simulink model "TSR_Scenario_005_cs"  (Simulink 7.6)
settings: Solver=ode5  SolverMode=SingleTasking  StartTime=0.0  StopTime=inf  FixedStep=1/100  RelTol=1e-3
PreLoadFcn:
  | check_NUTS_defined;
  | 
  | assignin('base',['model_closed_' bdroot],false);
  | 
  | addpath('../includes_TSR/includes_TSR');
  | 
  | switch version('-release')
  | case {'2010a', '2010b', '2011a'}
  | addpath('../includes_TSR/ML2010b');
  | otherwise
  | addpath('../includes_TSR/ML2011b');
  | end
InitFcn:
  | PreScanExperimentInitFcn(bdroot)
StopFcn:
  | PreScanExperimentStopFcn(bdroot)
undefined symbols (not defined in the file): DiscIntNeverNeededParam, InitImageOutputDir, x_ini_VRML, y_ini_VRML, z_ini_VRML
libraries: PreScanSimulinkLibrary x97, simulink x59, PreScanUsefulBlocks x33, simulink_extras x30, simulink_need_slupdate x1
(part 23 of 26)

== system TSR_Scenario_005_cs/Sphere_1/Dynamics_Empty/MuxState ==
  note: mode: Dynamics
  note: mode: Positioning
  note: mode: Dynamics
      | VelVEHICLE
      | wrt VEH
  note: AnglesVEHICLE
      | wrtGLOB
  note: For state mode 'Dynamic' positions
      | are not taken into account, as they
      | are computed by integrating velocity.
      | Click annotation for Help.
  x [m] : Inport  SampleTime=TimeStep
  y [m] : Inport  Port=2  SampleTime=TimeStep
  z [m] : Inport  Port=3  SampleTime=TimeStep
  dx [m/s] : Inport  Port=4  SampleTime=TimeStep
  dy [m/s] : Inport  Port=5  SampleTime=TimeStep
  dz [m/s] : Inport  Port=6  SampleTime=TimeStep
  R [rad] : Inport  Port=7  SampleTime=TimeStep
  P [rad] : Inport  Port=8  SampleTime=TimeStep
  Y [rad] : Inport  Port=9  SampleTime=TimeStep
  Constant : Constant  DisableCoverage=on  Value=positioningmode
  Discrete-Time Integrator : DiscreteIntegrator  SampleTime=TimeStep  ICPrevOutput=DiscIntNeverNeededParam  ICPrevScaledInput=DiscIntNeverNeededParam
  Ground : Ground
  Ground1 : Ground
  Mux1 : Mux  Inputs=[3 3]
  Mux2 : Mux  Inputs=3
  Mux3 : Mux  Inputs=3
  Mux4 : Mux  Inputs=3
  Mux5 : Mux  Inputs=[3 3]
  Mux6 : Mux  Inputs=[6 3]
  Switch : Switch  Threshold=0.5
  Transformation_BODY2GLOBAL : PreScanSimulinkLibrary/Simple_Vehicle_Dynamics/Chassis/MuxDynState/Transformation_BODY2GLOBAL  SystemSampleTime=-1
  mux3 : Mux  Inputs=3
  state : Outport
  wires:
  Constant/1 -> Switch/2
  Mux6/1 -> state/1
  Mux4/1 -> Mux1/1
  Mux5/1 -> Switch/3
  Mux1/1 -> Switch/1
  Switch/1 -> Mux6/1  "PosVeh wrt  GLOB"
  P [rad]/1 -> Mux3/2
  R [rad]/1 -> Mux3/1
  dz [m/s]/1 -> Mux2/3
  dy [m/s]/1 -> Mux2/2
  dx [m/s]/1 -> Mux2/1
  z [m]/1 -> Mux4/3
  y [m]/1 -> Mux4/2
  x [m]/1 -> Mux4/1
  Ground1/1 -> mux3/2  "pitch"
  Y [rad]/1 -> mux3/3, Mux3/3  "yaw"
  Mux3/1 -> Mux6/2
  Transformation_BODY2GLOBAL/1 -> Discrete-Time Integrator/1, Mux5/2  "VelVEH wrt  GLOB"
  mux3/1 -> Transformation_BODY2GLOBAL/2
  Ground/1 -> mux3/1  "roll"
  Mux2/1 -> Mux1/2, Transformation_BODY2GLOBAL/1
  Discrete-Time Integrator/1 -> Mux5/1  "PosVEH wrt  GLOB"

== system TSR_Scenario_005_cs/Sphere_1/SELF_Demux/compas converter ==
  rotz [rad] : Inport
  cart2compas : Subsystem
  rad2deg : Gain  Gain=180/pi
  heading [deg] : Outport
  wires:
  cart2compas/1(heading [deg]) -> heading [deg]/1
  rotz [rad]/1 -> rad2deg/1
  rad2deg/1 -> cart2compas/1(Angle [deg])

== system TSR_Scenario_005_cs/Toyota_Previa_1/Dynamics_Empty/MuxState ==
  note: mode: Dynamics
  note: mode: Positioning
  note: mode: Dynamics
      | VelVEHICLE
      | wrt VEH
  note: AnglesVEHICLE
      | wrtGLOB
  note: For state mode 'Dynamic' positions
      | are not taken into account, as they
      | are computed by integrating velocity.
      | Click annotation for Help.
  x [m] : Inport  SampleTime=TimeStep
  y [m] : Inport  Port=2  SampleTime=TimeStep
  z [m] : Inport  Port=3  SampleTime=TimeStep
  dx [m/s] : Inport  Port=4  SampleTime=TimeStep
  dy [m/s] : Inport  Port=5  SampleTime=TimeStep
  dz [m/s] : Inport  Port=6  SampleTime=TimeStep
  R [rad] : Inport  Port=7  SampleTime=TimeStep
  P [rad] : Inport  Port=8  SampleTime=TimeStep
  Y [rad] : Inport  Port=9  SampleTime=TimeStep
  Constant : Constant  DisableCoverage=on  Value=positioningmode
  Discrete-Time Integrator : DiscreteIntegrator  SampleTime=TimeStep  ICPrevOutput=DiscIntNeverNeededParam  ICPrevScaledInput=DiscIntNeverNeededParam
  Ground : Ground
  Ground1 : Ground
  Mux1 : Mux  Inputs=[3 3]
  Mux2 : Mux  Inputs=3
  Mux3 : Mux  Inputs=3
  Mux4 : Mux  Inputs=3
  Mux5 : Mux  Inputs=[3 3]
  Mux6 : Mux  Inputs=[6 3]
  Switch : Switch  Threshold=0.5
  Transformation_BODY2GLOBAL : PreScanSimulinkLibrary/Simple_Vehicle_Dynamics/Chassis/MuxDynState/Transformation_BODY2GLOBAL  SystemSampleTime=-1
  mux3 : Mux  Inputs=3
  state : Outport
  wires:
  Constant/1 -> Switch/2
  Mux6/1 -> state/1
  Mux4/1 -> Mux1/1
  Mux5/1 -> Switch/3
  Mux1/1 -> Switch/1
  Switch/1 -> Mux6/1  "PosVeh wrt  GLOB"
  P [rad]/1 -> Mux3/2
  R [rad]/1 -> Mux3/1
  dz [m/s]/1 -> Mux2/3
  dy [m/s]/1 -> Mux2/2
  dx [m/s]/1 -> Mux2/1
  z [m]/1 -> Mux4/3
  y [m]/1 -> Mux4/2
  x [m]/1 -> Mux4/1
  Ground1/1 -> mux3/2  "pitch"
  Y [rad]/1 -> mux3/3, Mux3/3  "yaw"
  Mux3/1 -> Mux6/2
  Transformation_BODY2GLOBAL/1 -> Discrete-Time Integrator/1, Mux5/2  "VelVEH wrt  GLOB"
  mux3/1 -> Transformation_BODY2GLOBAL/2
  Ground/1 -> mux3/1  "roll"
  Mux2/1 -> Mux1/2, Transformation_BODY2GLOBAL/1
  Discrete-Time Integrator/1 -> Mux5/1  "PosVEH wrt  GLOB"

== system TSR_Scenario_005_cs/Toyota_Previa_1/SELF_Demux/compas converter ==
  rotz [rad] : Inport
  cart2compas : Subsystem
  rad2deg : Gain  Gain=180/pi
  heading [deg] : Outport
  wires:
  cart2compas/1(heading [deg]) -> heading [deg]/1
  rotz [rad]/1 -> rad2deg/1
  rad2deg/1 -> cart2compas/1(Angle [deg])

== system TSR_Scenario_005_cs/Toyota_Previa_2/Dynamics_Empty/MuxState ==
  note: mode: Dynamics
  note: mode: Positioning
  note: mode: Dynamics
      | VelVEHICLE
      | wrt VEH
  note: AnglesVEHICLE
      | wrtGLOB
  note: For state mode 'Dynamic' positions
      | are not taken into account, as they
      | are computed by integrating velocity.
      | Click annotation for Help.
  x [m] : Inport  SampleTime=TimeStep
  y [m] : Inport  Port=2  SampleTime=TimeStep
  z [m] : Inport  Port=3  SampleTime=TimeStep
  dx [m/s] : Inport  Port=4  SampleTime=TimeStep
  dy [m/s] : Inport  Port=5  SampleTime=TimeStep
  dz [m/s] : Inport  Port=6  SampleTime=TimeStep
  R [rad] : Inport  Port=7  SampleTime=TimeStep
  P [rad] : Inport  Port=8  SampleTime=TimeStep
  Y [rad] : Inport  Port=9  SampleTime=TimeStep
  Constant : Constant  DisableCoverage=on  Value=positioningmode
  Discrete-Time Integrator : DiscreteIntegrator  SampleTime=TimeStep  ICPrevOutput=DiscIntNeverNeededParam  ICPrevScaledInput=DiscIntNeverNeededParam
  Ground : Ground
  Ground1 : Ground
  Mux1 : Mux  Inputs=[3 3]
  Mux2 : Mux  Inputs=3
  Mux3 : Mux  Inputs=3
  Mux4 : Mux  Inputs=3
  Mux5 : Mux  Inputs=[3 3]
  Mux6 : Mux  Inputs=[6 3]
  Switch : Switch  Threshold=0.5
  Transformation_BODY2GLOBAL : PreScanSimulinkLibrary/Simple_Vehicle_Dynamics/Chassis/MuxDynState/Transformation_BODY2GLOBAL  SystemSampleTime=-1
  mux3 : Mux  Inputs=3
  state : Outport
  wires:
  Constant/1 -> Switch/2
  Mux6/1 -> state/1
  Mux4/1 -> Mux1/1
  Mux5/1 -> Switch/3
  Mux1/1 -> Switch/1
  Switch/1 -> Mux6/1  "PosVeh wrt  GLOB"
  P [rad]/1 -> Mux3/2
  R [rad]/1 -> Mux3/1
  dz [m/s]/1 -> Mux2/3
  dy [m/s]/1 -> Mux2/2
  dx [m/s]/1 -> Mux2/1
  z [m]/1 -> Mux4/3
  y [m]/1 -> Mux4/2
  x [m]/1 -> Mux4/1
  Ground1/1 -> mux3/2  "pitch"
  Y [rad]/1 -> mux3/3, Mux3/3  "yaw"
  Mux3/1 -> Mux6/2
  Transformation_BODY2GLOBAL/1 -> Discrete-Time Integrator/1, Mux5/2  "VelVEH wrt  GLOB"
  mux3/1 -> Transformation_BODY2GLOBAL/2
  Ground/1 -> mux3/1  "roll"
  Mux2/1 -> Mux1/2, Transformation_BODY2GLOBAL/1
  Discrete-Time Integrator/1 -> Mux5/1  "PosVEH wrt  GLOB"

== system TSR_Scenario_005_cs/Toyota_Previa_2/SELF_Demux/compas converter ==
  rotz [rad] : Inport
  cart2compas : Subsystem
  rad2deg : Gain  Gain=180/pi
  heading [deg] : Outport
  wires:
  cart2compas/1(heading [deg]) -> heading [deg]/1
  rotz [rad]/1 -> rad2deg/1
  rad2deg/1 -> cart2compas/1(Angle [deg])

== system TSR_Scenario_005_cs/Toyota_Previa_3/Dynamics_Empty/MuxState ==
  note: mode: Dynamics
  note: mode: Positioning
  note: mode: Dynamics
      | VelVEHICLE
      | wrt VEH
  note: AnglesVEHICLE
      | wrtGLOB
  note: For state mode 'Dynamic' positions
      | are not taken into account, as they
      | are computed by integrating velocity.
      | Click annotation for Help.
  x [m] : Inport  SampleTime=TimeStep
  y [m] : Inport  Port=2  SampleTime=TimeStep
  z [m] : Inport  Port=3  SampleTime=TimeStep
  dx [m/s] : Inport  Port=4  SampleTime=TimeStep
  dy [m/s] : Inport  Port=5  SampleTime=TimeStep
  dz [m/s] : Inport  Port=6  SampleTime=TimeStep
  R [rad] : Inport  Port=7  SampleTime=TimeStep
  P [rad] : Inport  Port=8  SampleTime=TimeStep
  Y [rad] : Inport  Port=9  SampleTime=TimeStep
  Constant : Constant  DisableCoverage=on  Value=positioningmode
  Discrete-Time Integrator : DiscreteIntegrator  SampleTime=TimeStep  ICPrevOutput=DiscIntNeverNeededParam  ICPrevScaledInput=DiscIntNeverNeededParam
  Ground : Ground
  Ground1 : Ground
  Mux1 : Mux  Inputs=[3 3]
  Mux2 : Mux  Inputs=3
  Mux3 : Mux  Inputs=3
  Mux4 : Mux  Inputs=3
  Mux5 : Mux  Inputs=[3 3]
  Mux6 : Mux  Inputs=[6 3]
  Switch : Switch  Threshold=0.5
  Transformation_BODY2GLOBAL : PreScanSimulinkLibrary/Simple_Vehicle_Dynamics/Chassis/MuxDynState/Transformation_BODY2GLOBAL  SystemSampleTime=-1
  mux3 : Mux  Inputs=3
  state : Outport
  wires:
  Constant/1 -> Switch/2
  Mux6/1 -> state/1
  Mux4/1 -> Mux1/1
  Mux5/1 -> Switch/3
  Mux1/1 -> Switch/1
  Switch/1 -> Mux6/1  "PosVeh wrt  GLOB"
  P [rad]/1 -> Mux3/2
  R [rad]/1 -> Mux3/1
  dz [m/s]/1 -> Mux2/3
  dy [m/s]/1 -> Mux2/2
  dx [m/s]/1 -> Mux2/1
  z [m]/1 -> Mux4/3
  y [m]/1 -> Mux4/2
  x [m]/1 -> Mux4/1
  Ground1/1 -> mux3/2  "pitch"
  Y [rad]/1 -> mux3/3, Mux3/3  "yaw"
  Mux3/1 -> Mux6/2
  Transformation_BODY2GLOBAL/1 -> Discrete-Time Integrator/1, Mux5/2  "VelVEH wrt  GLOB"
  mux3/1 -> Transformation_BODY2GLOBAL/2
  Ground/1 -> mux3/1  "roll"
  Mux2/1 -> Mux1/2, Transformation_BODY2GLOBAL/1
  Discrete-Time Integrator/1 -> Mux5/1  "PosVEH wrt  GLOB"

== system TSR_Scenario_005_cs/Toyota_Previa_3/SELF_Demux/compas converter ==
  rotz [rad] : Inport
  cart2compas : Subsystem
  rad2deg : Gain  Gain=180/pi
  heading [deg] : Outport
  wires:
  cart2compas/1(heading [deg]) -> heading [deg]/1
  rotz [rad]/1 -> rad2deg/1
  rad2deg/1 -> cart2compas/1(Angle [deg])

== system TSR_Scenario_005_cs/Toyota_Previa_4/Dynamics_Empty/MuxState ==
  note: mode: Dynamics
  note: mode: Positioning
  note: mode: Dynamics
      | VelVEHICLE
      | wrt VEH
  note: AnglesVEHICLE
      | wrtGLOB
  note: For state mode 'Dynamic' positions
      | are not taken into account, as they
      | are computed by integrating velocity.
      | Click annotation for Help.
  x [m] : Inport  SampleTime=TimeStep
  y [m] : Inport  Port=2  SampleTime=TimeStep
  z [m] : Inport  Port=3  SampleTime=TimeStep
  dx [m/s] : Inport  Port=4  SampleTime=TimeStep
  dy [m/s] : Inport  Port=5  SampleTime=TimeStep
  dz [m/s] : Inport  Port=6  SampleTime=TimeStep
  R [rad] : Inport  Port=7  SampleTime=TimeStep
  P [rad] : Inport  Port=8  SampleTime=TimeStep
  Y [rad] : Inport  Port=9  SampleTime=TimeStep
  Constant : Constant  DisableCoverage=on  Value=positioningmode
  Discrete-Time Integrator : DiscreteIntegrator  SampleTime=TimeStep  ICPrevOutput=DiscIntNeverNeededParam  ICPrevScaledInput=DiscIntNeverNeededParam
  Ground : Ground
  Ground1 : Ground
  Mux1 : Mux  Inputs=[3 3]
  Mux2 : Mux  Inputs=3
  Mux3 : Mux  Inputs=3
  Mux4 : Mux  Inputs=3
  Mux5 : Mux  Inputs=[3 3]
  Mux6 : Mux  Inputs=[6 3]
  Switch : Switch  Threshold=0.5
  Transformation_BODY2GLOBAL : PreScanSimulinkLibrary/Simple_Vehicle_Dynamics/Chassis/MuxDynState/Transformation_BODY2GLOBAL  SystemSampleTime=-1
  mux3 : Mux  Inputs=3
  state : Outport
  wires:
  Constant/1 -> Switch/2
  Mux6/1 -> state/1
  Mux4/1 -> Mux1/1
  Mux5/1 -> Switch/3
  Mux1/1 -> Switch/1
  Switch/1 -> Mux6/1  "PosVeh wrt  GLOB"
  P [rad]/1 -> Mux3/2
  R [rad]/1 -> Mux3/1
  dz [m/s]/1 -> Mux2/3
  dy [m/s]/1 -> Mux2/2
  dx [m/s]/1 -> Mux2/1
  z [m]/1 -> Mux4/3
  y [m]/1 -> Mux4/2
  x [m]/1 -> Mux4/1
  Ground1/1 -> mux3/2  "pitch"
  Y [rad]/1 -> mux3/3, Mux3/3  "yaw"
  Mux3/1 -> Mux6/2
  Transformation_BODY2GLOBAL/1 -> Discrete-Time Integrator/1, Mux5/2  "VelVEH wrt  GLOB"
  mux3/1 -> Transformation_BODY2GLOBAL/2
  Ground/1 -> mux3/1  "roll"
  Mux2/1 -> Mux1/2, Transformation_BODY2GLOBAL/1
  Discrete-Time Integrator/1 -> Mux5/1  "PosVEH wrt  GLOB"

== system TSR_Scenario_005_cs/Toyota_Previa_4/SELF_Demux/compas converter ==
  rotz [rad] : Inport
  cart2compas : Subsystem
  rad2deg : Gain  Gain=180/pi
  heading [deg] : Outport
  wires:
  cart2compas/1(heading [deg]) -> heading [deg]/1
  rotz [rad]/1 -> rad2deg/1
  rad2deg/1 -> cart2compas/1(Angle [deg])

== system TSR_Scenario_005_cs/Toyota_Yaris_1/SELF_Demux/compas converter ==
  rotz [rad] : Inport
  cart2compas : Subsystem
  rad2deg : Gain  Gain=180/pi
  heading [deg] : Outport
  wires:
  cart2compas/1(heading [deg]) -> heading [deg]/1
  rotz [rad]/1 -> rad2deg/1
  rad2deg/1 -> cart2compas/1(Angle [deg])

== system TSR_Scenario_005_cs/Toyota_Yaris_1/Trajectories/Traj2State1 ==
  Traj Org : Inport
  Offset : Inport  Port=2
  Add2 : Sum
  ApplyRotation : PreScanSimulinkLibrary/ApplyRotation  SystemSampleTime=-1
  ApplyRotation1 : PreScanSimulinkLibrary/ApplyRotation  SystemSampleTime=-1
  ApplyRotation2 : PreScanSimulinkLibrary/ApplyRotation  SystemSampleTime=-1
  Constant : Constant  Value=zeros(12,1)
  Mux : Mux  Inputs=6
  Mux1 : Mux  Inputs=2
  Mux{2,4..9} (7 alike) : Mux  Inputs=3
  Mux3 : Mux  Inputs=2
  Selector : Selector  InputPortWidth=6  IndexOptions=Index vector (dialog)  Indices=[4 5 6]  OutputSizes=1
  Selector1 : Selector  InputPortWidth=21  IndexOptions=Index vector (dialog)  Indices=[5]  OutputSizes=1
  Selector10 : Selector  InputPortWidth=21  IndexOptions=Index vector (dialog)  Indices=[2]  OutputSizes=1
  Selector11 : Selector  InputPortWidth=21  IndexOptions=Index vector (dialog)  Indices=[3]  OutputSizes=1
  Selector12 : Selector  InputPortWidth=21  IndexOptions=Index vector (dialog)  Indices=[1]  OutputSizes=1
  Selector13 : Selector  InputPortWidth=21  IndexOptions=Index vector (dialog)  Indices=[8]  OutputSizes=1
  Selector14 : Selector  InputPortWidth=21  IndexOptions=Index vector (dialog)  Indices=[9]  OutputSizes=1
  Selector15 : Selector  InputPortWidth=21  IndexOptions=Index vector (dialog)  Indices=[7]  OutputSizes=1
  Selector16 : Selector  InputPortWidth=21  IndexOptions=Index vector (dialog)  Indices=[14]  OutputSizes=1
  Selector17 : Selector  InputPortWidth=21  IndexOptions=Index vector (dialog)  Indices=[15]  OutputSizes=1
  Selector18 : Selector  InputPortWidth=21  IndexOptions=Index vector (dialog)  Indices=[13]  OutputSizes=1
  Selector19 : Selector  InputPortWidth=21  IndexOptions=Index vector (dialog)  Indices=[20]  OutputSizes=1
  Selector2 : Selector  InputPortWidth=21  IndexOptions=Index vector (dialog)  Indices=[6]  OutputSizes=1
  Selector20 : Selector  InputPortWidth=21  IndexOptions=Index vector (dialog)  Indices=[21]  OutputSizes=1
  Selector21 : Selector  InputPortWidth=21  IndexOptions=Index vector (dialog)  Indices=[19]  OutputSizes=1
  Selector3 : Selector  InputPortWidth=21  IndexOptions=Index vector (dialog)  Indices=[12]  OutputSizes=1
  Selector4 : Selector  InputPortWidth=21  IndexOptions=Index vector (dialog)  Indices=[17]  OutputSizes=1
  Selector5 : Selector  InputPortWidth=21  IndexOptions=Index vector (dialog)  Indices=[18]  OutputSizes=1
  Selector6 : Selector  InputPortWidth=21  IndexOptions=Index vector (dialog)  Indices=[16]  OutputSizes=1
  Selector7 : Selector  InputPortWidth=21  IndexOptions=Index vector (dialog)  Indices=[11]  OutputSizes=1
  Selector8 : Selector  InputPortWidth=21  IndexOptions=Index vector (dialog)  Indices=[10]  OutputSizes=1
  Selector9 : Selector  InputPortWidth=21  IndexOptions=Index vector (dialog)  Indices=[4]  OutputSizes=1
  Traj2State2 : Subsystem
  state : Outport
  wires:
  Mux9/1 -> ApplyRotation2/2, ApplyRotation1/2, ApplyRotation/2
  Selector20/1 -> Mux9/3
  Selector19/1 -> Mux9/2
  Selector21/1 -> Mux9/1
  Selector5/1 -> Mux8/3
  Selector4/1 -> Mux8/2
  Selector6/1 -> Mux8/1
  Mux8/1 -> Mux/6
  Mux6/1 -> ApplyRotation2/1
  Mux7/1 -> Mux/4
  ApplyRotation2/1 -> Mux/5
  Selector3/1 -> Mux7/3
  Selector7/1 -> Mux7/2
  Selector8/1 -> Mux7/1
  Mux4/1 -> Mux/2
  Mux5/1 -> ApplyRotation1/1
  ApplyRotation1/1 -> Mux/3
  Mux2/1 -> ApplyRotation/1
  ApplyRotation/1 -> Mux/1
  Selector2/1 -> Mux4/3
  Selector1/1 -> Mux4/2
  Selector9/1 -> Mux4/1
  Selector17/1 -> Mux6/3
  Selector16/1 -> Mux6/2
  Selector18/1 -> Mux6/1
  Selector14/1 -> Mux5/3
  Selector13/1 -> Mux5/2
  Selector15/1 -> Mux5/1
  Selector11/1 -> Mux2/3
  Selector10/1 -> Mux2/2
  Selector12/1 -> Mux2/1
  Mux3/1 -> Selector8/1, Selector7/1, Selector3/1, Selector18/1, Selector16/1, Selector17/1, Selector6/1, Selector20/1, Selector19/1, Selector21/1, Selector5/1, Selector4/1, Selector14/1, Selector13/1, Selector15/1, Selector2/1, Selector1/1, Selector9/1, Selector12/1, Selector10/1, Selector11/1
  Mux1/1 -> Add2/2
  Constant/1 -> Mux1/2
  Add2/1 -> Traj2State2/1(traj)
  Selector/1 -> Mux3/2
  Traj Org/1 -> Mux3/1
  Offset/1 -> Mux1/1, Selector/1
  Mux/1 -> Add2/1
  Traj2State2/1(state) -> state/1

== system TSR_Scenario_005_cs/Toyota_Yaris_1/Trajectories/Trajectory_5 ==
  From Workspace : FromWorkspace  VariableName=trajectory  SampleTime=TimeStep  OutputAfterFinalValue=Holding final value
  From Workspace1 : FromWorkspace  VariableName=trajectory  SampleTime=TimeStep  OutputAfterFinalValue=Setting to zero
  Mux : Mux  Inputs=2
  Selector1 : Selector  InputPortWidth=24  IndexOptions=Index vector (dialog)  Indices=[7:18]  OutputSizes=1
  Selector9 : Selector  InputPortWidth=24  IndexOptions=Index vector (dialog)  Indices=[1:6]  OutputSizes=1
  trajectory : Outport
  wires:
  Selector1/1 -> Mux/2
  Selector9/1 -> Mux/1
  Mux/1 -> trajectory/1
  From Workspace1/1 -> Selector1/1
  From Workspace/1 -> Selector9/1

== system TSR_Scenario_005_cs/Toyota_Yaris_2/Dynamics_Empty/MuxState ==
  note: mode: Dynamics
  note: mode: Positioning
  note: mode: Dynamics
      | VelVEHICLE
      | wrt VEH
  note: AnglesVEHICLE
      | wrtGLOB
  note: For state mode 'Dynamic' positions
      | are not taken into account, as they
      | are computed by integrating velocity.
      | Click annotation for Help.
  x [m] : Inport  SampleTime=TimeStep
  y [m] : Inport  Port=2  SampleTime=TimeStep
  z [m] : Inport  Port=3  SampleTime=TimeStep
  dx [m/s] : Inport  Port=4  SampleTime=TimeStep
  dy [m/s] : Inport  Port=5  SampleTime=TimeStep
  dz [m/s] : Inport  Port=6  SampleTime=TimeStep
  R [rad] : Inport  Port=7  SampleTime=TimeStep
  P [rad] : Inport  Port=8  SampleTime=TimeStep
  Y [rad] : Inport  Port=9  SampleTime=TimeStep
  Constant : Constant  DisableCoverage=on  Value=positioningmode
  Discrete-Time Integrator : DiscreteIntegrator  SampleTime=TimeStep  ICPrevOutput=DiscIntNeverNeededParam  ICPrevScaledInput=DiscIntNeverNeededParam
  Ground : Ground
  Ground1 : Ground
  Mux1 : Mux  Inputs=[3 3]
  Mux2 : Mux  Inputs=3
  Mux3 : Mux  Inputs=3
  Mux4 : Mux  Inputs=3
  Mux5 : Mux  Inputs=[3 3]
  Mux6 : Mux  Inputs=[6 3]
  Switch : Switch  Threshold=0.5
  Transformation_BODY2GLOBAL : PreScanSimulinkLibrary/Simple_Vehicle_Dynamics/Chassis/MuxDynState/Transformation_BODY2GLOBAL  SystemSampleTime=-1
  mux3 : Mux  Inputs=3
  state : Outport
  wires:
  Constant/1 -> Switch/2
  Mux6/1 -> state/1
  Mux4/1 -> Mux1/1
  Mux5/1 -> Switch/3
  Mux1/1 -> Switch/1
  Switch/1 -> Mux6/1  "PosVeh wrt  GLOB"
  P [rad]/1 -> Mux3/2
  R [rad]/1 -> Mux3/1
  dz [m/s]/1 -> Mux2/3
  dy [m/s]/1 -> Mux2/2
  dx [m/s]/1 -> Mux2/1
  z [m]/1 -> Mux4/3
  y [m]/1 -> Mux4/2
  x [m]/1 -> Mux4/1
  Ground1/1 -> mux3/2  "pitch"
  Y [rad]/1 -> mux3/3, Mux3/3  "yaw"
  Mux3/1 -> Mux6/2
  Transformation_BODY2GLOBAL/1 -> Discrete-Time Integrator/1, Mux5/2  "VelVEH wrt  GLOB"
  mux3/1 -> Transformation_BODY2GLOBAL/2
  Ground/1 -> mux3/1  "roll"
  Mux2/1 -> Mux1/2, Transformation_BODY2GLOBAL/1
  Discrete-Time Integrator/1 -> Mux5/1  "PosVEH wrt  GLOB"

== system TSR_Scenario_005_cs/Toyota_Yaris_2/SELF_Demux/compas converter ==
  rotz [rad] : Inport
  cart2compas : Subsystem
  rad2deg : Gain  Gain=180/pi
  heading [deg] : Outport
  wires:
  cart2compas/1(heading [deg]) -> heading [deg]/1
  rotz [rad]/1 -> rad2deg/1
  rad2deg/1 -> cart2compas/1(Angle [deg])

== system TSR_Scenario_005_cs/VISUBrakeLightL_Audi_A8_1/scaler/scale B ==
  color : Inport
  scale : Inport  Port=2
  Product : Product  RndMeth=Zero
  Switch : Switch  Threshold=255
  scaled color : Outport
  wires:
  color/1 -> Product/2, Switch/2, Switch/3
  Switch/1 -> scaled color/1
  Product/1 -> Switch/1
  scale/1 -> Product/1

== system TSR_Scenario_005_cs/VISUBrakeLightL_Audi_A8_1/scaler/scale G ==
  color : Inport
  scale : Inport  Port=2
  Product : Product  RndMeth=Zero
  Switch : Switch  Threshold=255
  scaled color : Outport
  wires:
  color/1 -> Product/2, Switch/2, Switch/3
  Switch/1 -> scaled color/1
  Product/1 -> Switch/1
  scale/1 -> Product/1

== system TSR_Scenario_005_cs/VISUBrakeLightL_Audi_A8_1/scaler/scale R ==
  color : Inport
  scale : Inport  Port=2
  Product : Product  RndMeth=Zero
  Switch : Switch  Threshold=255
  scaled color : Outport
  wires:
  scale/1 -> Product/1
  Product/1 -> Switch/1
  Switch/1 -> scaled color/1
  color/1 -> Switch/3, Switch/2, Product/2

== system TSR_Scenario_005_cs/VISUBrakeLightR_Audi_A8_1/scaler/scale B ==
  color : Inport
  scale : Inport  Port=2
  Product : Product  RndMeth=Zero
  Switch : Switch  Threshold=255
  scaled color : Outport
  wires:
  color/1 -> Product/2, Switch/2, Switch/3
  Switch/1 -> scaled color/1
  Product/1 -> Switch/1
  scale/1 -> Product/1

== system TSR_Scenario_005_cs/VISUBrakeLightR_Audi_A8_1/scaler/scale G ==
  color : Inport
  scale : Inport  Port=2
  Product : Product  RndMeth=Zero
  Switch : Switch  Threshold=255
  scaled color : Outport
  wires:
  color/1 -> Product/2, Switch/2, Switch/3
  Switch/1 -> scaled color/1
  Product/1 -> Switch/1
  scale/1 -> Product/1

== system TSR_Scenario_005_cs/VISUBrakeLightR_Audi_A8_1/scaler/scale R ==
  color : Inport
  scale : Inport  Port=2
  Product : Product  RndMeth=Zero
  Switch : Switch  Threshold=255
  scaled color : Outport
  wires:
  scale/1 -> Product/1
  Product/1 -> Switch/1
  Switch/1 -> scaled color/1
  color/1 -> Switch/3, Switch/2, Product/2

== system TSR_Scenario_005_cs/VISUHeadLightLeftHBLB_Audi_A8_1/scaler/scale B ==
  color : Inport
  scale : Inport  Port=2
  Product : Product  RndMeth=Zero
  Switch : Switch  Threshold=255
  scaled color : Outport
  wires:
  color/1 -> Product/2, Switch/2, Switch/3
  Switch/1 -> scaled color/1
  Product/1 -> Switch/1
  scale/1 -> Product/1

== system TSR_Scenario_005_cs/VISUHeadLightLeftHBLB_Audi_A8_1/scaler/scale G ==
  color : Inport
  scale : Inport  Port=2
  Product : Product  RndMeth=Zero
  Switch : Switch  Threshold=255
  scaled color : Outport
  wires:
  color/1 -> Product/2, Switch/2, Switch/3
  Switch/1 -> scaled color/1
  Product/1 -> Switch/1
  scale/1 -> Product/1

== system TSR_Scenario_005_cs/VISUHeadLightLeftHBLB_Audi_A8_1/scaler/scale R ==
  color : Inport
  scale : Inport  Port=2
  Product : Product  RndMeth=Zero
  Switch : Switch  Threshold=255
  scaled color : Outport
  wires:
  scale/1 -> Product/1
  Product/1 -> Switch/1
  Switch/1 -> scaled color/1
  color/1 -> Switch/3, Switch/2, Product/2

== system TSR_Scenario_005_cs/VISUHeadLightRightHBLB_Audi_A8_1/scaler/scale B ==
  color : Inport
  scale : Inport  Port=2
  Product : Product  RndMeth=Zero
  Switch : Switch  Threshold=255
  scaled color : Outport
  wires:
  color/1 -> Product/2, Switch/2, Switch/3
  Switch/1 -> scaled color/1
  Product/1 -> Switch/1
  scale/1 -> Product/1

== system TSR_Scenario_005_cs/VISUHeadLightRightHBLB_Audi_A8_1/scaler/scale G ==
  color : Inport
  scale : Inport  Port=2
  Product : Product  RndMeth=Zero
  Switch : Switch  Threshold=255
  scaled color : Outport
  wires:
  color/1 -> Product/2, Switch/2, Switch/3
  Switch/1 -> scaled color/1
  Product/1 -> Switch/1
  scale/1 -> Product/1

== system TSR_Scenario_005_cs/VISUHeadLightRightHBLB_Audi_A8_1/scaler/scale R ==
  color : Inport
  scale : Inport  Port=2
  Product : Product  RndMeth=Zero
  Switch : Switch  Threshold=255
  scaled color : Outport
  wires:
  scale/1 -> Product/1
  Product/1 -> Switch/1
  Switch/1 -> scaled color/1
  color/1 -> Switch/3, Switch/2, Product/2

== system TSR_Scenario_005_cs/VISULightPostLight_Lightpost_1/scaler/scale B ==
  color : Inport
  scale : Inport  Port=2
  Product : Product  RndMeth=Zero
  Switch : Switch  Threshold=255
  scaled color : Outport
  wires:
  color/1 -> Product/2, Switch/2, Switch/3
  Switch/1 -> scaled color/1
  Product/1 -> Switch/1
  scale/1 -> Product/1

== system TSR_Scenario_005_cs/VISULightPostLight_Lightpost_1/scaler/scale G ==
  color : Inport
  scale : Inport  Port=2
  Product : Product  RndMeth=Zero
  Switch : Switch  Threshold=255
  scaled color : Outport
  wires:
  color/1 -> Product/2, Switch/2, Switch/3
  Switch/1 -> scaled color/1
  Product/1 -> Switch/1
  scale/1 -> Product/1

== system TSR_Scenario_005_cs/VISULightPostLight_Lightpost_1/scaler/scale R ==
  color : Inport
  scale : Inport  Port=2
  Product : Product  RndMeth=Zero
  Switch : Switch  Threshold=255
  scaled color : Outport
  wires:
  scale/1 -> Product/1
  Product/1 -> Switch/1
  Switch/1 -> scaled color/1
  color/1 -> Switch/3, Switch/2, Product/2

== system TSR_Scenario_005_cs/VISULightPostLight_Lightpost_11/scaler/scale B ==
  color : Inport
  scale : Inport  Port=2
  Product : Product  RndMeth=Zero
  Switch : Switch  Threshold=255
  scaled color : Outport
  wires:
  color/1 -> Product/2, Switch/2, Switch/3
  Switch/1 -> scaled color/1
  Product/1 -> Switch/1
  scale/1 -> Product/1

== system TSR_Scenario_005_cs/VISULightPostLight_Lightpost_11/scaler/scale G ==
  color : Inport
  scale : Inport  Port=2
  Product : Product  RndMeth=Zero
  Switch : Switch  Threshold=255
  scaled color : Outport
  wires:
  color/1 -> Product/2, Switch/2, Switch/3
  Switch/1 -> scaled color/1
  Product/1 -> Switch/1
  scale/1 -> Product/1

== system TSR_Scenario_005_cs/VISULightPostLight_Lightpost_11/scaler/scale R ==
  color : Inport
  scale : Inport  Port=2
  Product : Product  RndMeth=Zero
  Switch : Switch  Threshold=255
  scaled color : Outport
  wires:
  scale/1 -> Product/1
  Product/1 -> Switch/1
  Switch/1 -> scaled color/1
  color/1 -> Switch/3, Switch/2, Product/2

== system TSR_Scenario_005_cs/VISULightPostLight_Lightpost_12/scaler/scale B ==
  color : Inport
  scale : Inport  Port=2
  Product : Product  RndMeth=Zero
  Switch : Switch  Threshold=255
  scaled color : Outport
  wires:
  color/1 -> Product/2, Switch/2, Switch/3
  Switch/1 -> scaled color/1
  Product/1 -> Switch/1
  scale/1 -> Product/1

== system TSR_Scenario_005_cs/VISULightPostLight_Lightpost_12/scaler/scale G ==
  color : Inport
  scale : Inport  Port=2
  Product : Product  RndMeth=Zero
  Switch : Switch  Threshold=255
  scaled color : Outport
  wires:
  color/1 -> Product/2, Switch/2, Switch/3
  Switch/1 -> scaled color/1
  Product/1 -> Switch/1
  scale/1 -> Product/1

== system TSR_Scenario_005_cs/VISULightPostLight_Lightpost_12/scaler/scale R ==
  color : Inport
  scale : Inport  Port=2
  Product : Product  RndMeth=Zero
  Switch : Switch  Threshold=255
  scaled color : Outport
  wires:
  scale/1 -> Product/1
  Product/1 -> Switch/1
  Switch/1 -> scaled color/1
  color/1 -> Switch/3, Switch/2, Product/2

== system TSR_Scenario_005_cs/VISULightPostLight_Lightpost_2/scaler/scale B ==
  color : Inport
  scale : Inport  Port=2
  Product : Product  RndMeth=Zero
  Switch : Switch  Threshold=255
  scaled color : Outport
  wires:
  color/1 -> Product/2, Switch/2, Switch/3
  Switch/1 -> scaled color/1
  Product/1 -> Switch/1
  scale/1 -> Product/1

== system TSR_Scenario_005_cs/VISULightPostLight_Lightpost_2/scaler/scale G ==
  color : Inport
  scale : Inport  Port=2
  Product : Product  RndMeth=Zero
  Switch : Switch  Threshold=255
  scaled color : Outport
  wires:
  color/1 -> Product/2, Switch/2, Switch/3
  Switch/1 -> scaled color/1
  Product/1 -> Switch/1
  scale/1 -> Product/1

== system TSR_Scenario_005_cs/VISULightPostLight_Lightpost_2/scaler/scale R ==
  color : Inport
  scale : Inport  Port=2
  Product : Product  RndMeth=Zero
  Switch : Switch  Threshold=255
  scaled color : Outport
  wires:
  scale/1 -> Product/1
  Product/1 -> Switch/1
  Switch/1 -> scaled color/1
  color/1 -> Switch/3, Switch/2, Product/2

== system TSR_Scenario_005_cs/VISULightPostLight_Lightpost_25/scaler/scale B ==
  color : Inport
  scale : Inport  Port=2
  Product : Product  RndMeth=Zero
  Switch : Switch  Threshold=255
  scaled color : Outport
  wires:
  color/1 -> Product/2, Switch/2, Switch/3
  Switch/1 -> scaled color/1
  Product/1 -> Switch/1
  scale/1 -> Product/1

== system TSR_Scenario_005_cs/VISULightPostLight_Lightpost_25/scaler/scale G ==
  color : Inport
  scale : Inport  Port=2
  Product : Product  RndMeth=Zero
  Switch : Switch  Threshold=255
  scaled color : Outport
  wires:
  color/1 -> Product/2, Switch/2, Switch/3
  Switch/1 -> scaled color/1
  Product/1 -> Switch/1
  scale/1 -> Product/1

== system TSR_Scenario_005_cs/VISULightPostLight_Lightpost_25/scaler/scale R ==
  color : Inport
  scale : Inport  Port=2
  Product : Product  RndMeth=Zero
  Switch : Switch  Threshold=255
  scaled color : Outport
  wires:
  scale/1 -> Product/1
  Product/1 -> Switch/1
  Switch/1 -> scaled color/1
  color/1 -> Switch/3, Switch/2, Product/2

== system TSR_Scenario_005_cs/VISULightPostLight_Lightpost_27/scaler/scale B ==
  color : Inport
  scale : Inport  Port=2
  Product : Product  RndMeth=Zero
  Switch : Switch  Threshold=255
  scaled color : Outport
  wires:
  color/1 -> Product/2, Switch/2, Switch/3
  Switch/1 -> scaled color/1
  Product/1 -> Switch/1
  scale/1 -> Product/1

== system TSR_Scenario_005_cs/VISULightPostLight_Lightpost_27/scaler/scale G ==
  color : Inport
  scale : Inport  Port=2
  Product : Product  RndMeth=Zero
  Switch : Switch  Threshold=255
  scaled color : Outport
  wires:
  color/1 -> Product/2, Switch/2, Switch/3
  Switch/1 -> scaled color/1
  Product/1 -> Switch/1
  scale/1 -> Product/1

== system TSR_Scenario_005_cs/VISULightPostLight_Lightpost_27/scaler/scale R ==
  color : Inport
  scale : Inport  Port=2
  Product : Product  RndMeth=Zero
  Switch : Switch  Threshold=255
  scaled color : Outport
  wires:
  scale/1 -> Product/1
  Product/1 -> Switch/1
  Switch/1 -> scaled color/1
  color/1 -> Switch/3, Switch/2, Product/2

== system TSR_Scenario_005_cs/VISULightPostLight_Lightpost_3/scaler/scale B ==
  color : Inport
  scale : Inport  Port=2
  Product : Product  RndMeth=Zero
  Switch : Switch  Threshold=255
  scaled color : Outport
  wires:
  color/1 -> Product/2, Switch/2, Switch/3
  Switch/1 -> scaled color/1
  Product/1 -> Switch/1
  scale/1 -> Product/1

== system TSR_Scenario_005_cs/VISULightPostLight_Lightpost_3/scaler/scale G ==
  color : Inport
  scale : Inport  Port=2
  Product : Product  RndMeth=Zero
  Switch : Switch  Threshold=255
  scaled color : Outport
  wires:
  color/1 -> Product/2, Switch/2, Switch/3
  Switch/1 -> scaled color/1
  Product/1 -> Switch/1
  scale/1 -> Product/1

== system TSR_Scenario_005_cs/VISULightPostLight_Lightpost_3/scaler/scale R ==
  color : Inport
  scale : Inport  Port=2
  Product : Product  RndMeth=Zero
  Switch : Switch  Threshold=255
  scaled color : Outport
  wires:
  scale/1 -> Product/1
  Product/1 -> Switch/1
  Switch/1 -> scaled color/1
  color/1 -> Switch/3, Switch/2, Product/2

== system TSR_Scenario_005_cs/VISULightPostLight_Lightpost_34/scaler/scale B ==
  color : Inport
  scale : Inport  Port=2
  Product : Product  RndMeth=Zero
  Switch : Switch  Threshold=255
  scaled color : Outport
  wires:
  color/1 -> Product/2, Switch/2, Switch/3
  Switch/1 -> scaled color/1
  Product/1 -> Switch/1
  scale/1 -> Product/1

== system TSR_Scenario_005_cs/VISULightPostLight_Lightpost_34/scaler/scale G ==
  color : Inport
  scale : Inport  Port=2
  Product : Product  RndMeth=Zero
  Switch : Switch  Threshold=255
  scaled color : Outport
  wires:
  color/1 -> Product/2, Switch/2, Switch/3
  Switch/1 -> scaled color/1
  Product/1 -> Switch/1
  scale/1 -> Product/1

== system TSR_Scenario_005_cs/VISULightPostLight_Lightpost_34/scaler/scale R ==
  color : Inport
  scale : Inport  Port=2
  Product : Product  RndMeth=Zero
  Switch : Switch  Threshold=255
  scaled color : Outport
  wires:
  scale/1 -> Product/1
  Product/1 -> Switch/1
  Switch/1 -> scaled color/1
  color/1 -> Switch/3, Switch/2, Product/2

== system TSR_Scenario_005_cs/VISULightPostLight_Lightpost_36/scaler/scale B ==
  color : Inport
  scale : Inport  Port=2
  Product : Product  RndMeth=Zero
  Switch : Switch  Threshold=255
  scaled color : Outport
  wires:
  color/1 -> Product/2, Switch/2, Switch/3
  Switch/1 -> scaled color/1
  Product/1 -> Switch/1
  scale/1 -> Product/1

== system TSR_Scenario_005_cs/VISULightPostLight_Lightpost_36/scaler/scale G ==
  color : Inport
  scale : Inport  Port=2
  Product : Product  RndMeth=Zero
  Switch : Switch  Threshold=255
  scaled color : Outport
  wires:
  color/1 -> Product/2, Switch/2, Switch/3
  Switch/1 -> scaled color/1
  Product/1 -> Switch/1
  scale/1 -> Product/1

== system TSR_Scenario_005_cs/VISULightPostLight_Lightpost_36/scaler/scale R ==
  color : Inport
  scale : Inport  Port=2
  Product : Product  RndMeth=Zero
  Switch : Switch  Threshold=255
  scaled color : Outport
  wires:
  scale/1 -> Product/1
  Product/1 -> Switch/1
  Switch/1 -> scaled color/1
  color/1 -> Switch/3, Switch/2, Product/2

== system TSR_Scenario_005_cs/VISULightPostLight_Lightpost_38/scaler/scale B ==
  color : Inport
  scale : Inport  Port=2
  Product : Product  RndMeth=Zero
  Switch : Switch  Threshold=255
  scaled color : Outport
  wires:
  color/1 -> Product/2, Switch/2, Switch/3
  Switch/1 -> scaled color/1
  Product/1 -> Switch/1
  scale/1 -> Product/1

== system TSR_Scenario_005_cs/VISULightPostLight_Lightpost_38/scaler/scale G ==
  color : Inport
  scale : Inport  Port=2
  Product : Product  RndMeth=Zero
  Switch : Switch  Threshold=255
  scaled color : Outport
  wires:
  color/1 -> Product/2, Switch/2, Switch/3
  Switch/1 -> scaled color/1
  Product/1 -> Switch/1
  scale/1 -> Product/1

== system TSR_Scenario_005_cs/VISULightPostLight_Lightpost_38/scaler/scale R ==
  color : Inport
  scale : Inport  Port=2
  Product : Product  RndMeth=Zero
  Switch : Switch  Threshold=255
  scaled color : Outport
  wires:
  scale/1 -> Product/1
  Product/1 -> Switch/1
  Switch/1 -> scaled color/1
  color/1 -> Switch/3, Switch/2, Product/2

== system TSR_Scenario_005_cs/VISULightPostLight_Lightpost_4/scaler/scale B ==
  color : Inport
  scale : Inport  Port=2
  Product : Product  RndMeth=Zero
  Switch : Switch  Threshold=255
  scaled color : Outport
  wires:
  color/1 -> Product/2, Switch/2, Switch/3
  Switch/1 -> scaled color/1
  Product/1 -> Switch/1
  scale/1 -> Product/1

== system TSR_Scenario_005_cs/VISULightPostLight_Lightpost_4/scaler/scale G ==
  color : Inport
  scale : Inport  Port=2
  Product : Product  RndMeth=Zero
  Switch : Switch  Threshold=255
  scaled color : Outport
  wires:
  color/1 -> Product/2, Switch/2, Switch/3
  Switch/1 -> scaled color/1
  Product/1 -> Switch/1
  scale/1 -> Product/1

== system TSR_Scenario_005_cs/VISULightPostLight_Lightpost_4/scaler/scale R ==
  color : Inport
  scale : Inport  Port=2
  Product : Product  RndMeth=Zero
  Switch : Switch  Threshold=255
  scaled color : Outport
  wires:
  scale/1 -> Product/1
  Product/1 -> Switch/1
  Switch/1 -> scaled color/1
  color/1 -> Switch/3, Switch/2, Product/2

== system TSR_Scenario_005_cs/VISULightPostLight_Lightpost_40/scaler/scale B ==
  color : Inport
  scale : Inport  Port=2
  Product : Product  RndMeth=Zero
  Switch : Switch  Threshold=255
  scaled color : Outport
  wires:
  color/1 -> Product/2, Switch/2, Switch/3
  Switch/1 -> scaled color/1
  Product/1 -> Switch/1
  scale/1 -> Product/1

== system TSR_Scenario_005_cs/VISULightPostLight_Lightpost_40/scaler/scale G ==
  color : Inport
  scale : Inport  Port=2
  Product : Product  RndMeth=Zero
  Switch : Switch  Threshold=255
  scaled color : Outport
  wires:
  color/1 -> Product/2, Switch/2, Switch/3
  Switch/1 -> scaled color/1
  Product/1 -> Switch/1
  scale/1 -> Product/1

== system TSR_Scenario_005_cs/VISULightPostLight_Lightpost_40/scaler/scale R ==
  color : Inport
  scale : Inport  Port=2
  Product : Product  RndMeth=Zero
  Switch : Switch  Threshold=255
  scaled color : Outport
  wires:
  scale/1 -> Product/1
  Product/1 -> Switch/1
  Switch/1 -> scaled color/1
  color/1 -> Switch/3, Switch/2, Product/2

== system TSR_Scenario_005_cs/VISULightPostLight_Lightpost_42/scaler/scale B ==
  color : Inport
  scale : Inport  Port=2
  Product : Product  RndMeth=Zero
  Switch : Switch  Threshold=255
  scaled color : Outport
  wires:
  color/1 -> Product/2, Switch/2, Switch/3
  Switch/1 -> scaled color/1
  Product/1 -> Switch/1
  scale/1 -> Product/1

== system TSR_Scenario_005_cs/VISULightPostLight_Lightpost_42/scaler/scale G ==
  color : Inport
  scale : Inport  Port=2
  Product : Product  RndMeth=Zero
  Switch : Switch  Threshold=255
  scaled color : Outport
  wires:
  color/1 -> Product/2, Switch/2, Switch/3
  Switch/1 -> scaled color/1
  Product/1 -> Switch/1
  scale/1 -> Product/1

== system TSR_Scenario_005_cs/VISULightPostLight_Lightpost_42/scaler/scale R ==
  color : Inport
  scale : Inport  Port=2
  Product : Product  RndMeth=Zero
  Switch : Switch  Threshold=255
  scaled color : Outport
  wires:
  scale/1 -> Product/1
  Product/1 -> Switch/1
  Switch/1 -> scaled color/1
  color/1 -> Switch/3, Switch/2, Product/2

== system TSR_Scenario_005_cs/VISULightPostLight_Lightpost_44/scaler/scale B ==
  color : Inport
  scale : Inport  Port=2
  Product : Product  RndMeth=Zero
  Switch : Switch  Threshold=255
  scaled color : Outport
  wires:
  color/1 -> Product/2, Switch/2, Switch/3
  Switch/1 -> scaled color/1
  Product/1 -> Switch/1
  scale/1 -> Product/1

== system TSR_Scenario_005_cs/VISULightPostLight_Lightpost_44/scaler/scale G ==
  color : Inport
  scale : Inport  Port=2
  Product : Product  RndMeth=Zero
  Switch : Switch  Threshold=255
  scaled color : Outport
  wires:
  color/1 -> Product/2, Switch/2, Switch/3
  Switch/1 -> scaled color/1
  Product/1 -> Switch/1
  scale/1 -> Product/1

== system TSR_Scenario_005_cs/VISULightPostLight_Lightpost_44/scaler/scale R ==
  color : Inport
  scale : Inport  Port=2
  Product : Product  RndMeth=Zero
  Switch : Switch  Threshold=255
  scaled color : Outport
  wires:
  scale/1 -> Product/1
  Product/1 -> Switch/1
  Switch/1 -> scaled color/1
  color/1 -> Switch/3, Switch/2, Product/2

== system TSR_Scenario_005_cs/VISULightPostLight_Lightpost_5/scaler/scale B ==
  color : Inport
  scale : Inport  Port=2
  Product : Product  RndMeth=Zero
  Switch : Switch  Threshold=255
  scaled color : Outport
  wires:
  color/1 -> Product/2, Switch/2, Switch/3
  Switch/1 -> scaled color/1
  Product/1 -> Switch/1
  scale/1 -> Product/1

== system TSR_Scenario_005_cs/VISULightPostLight_Lightpost_5/scaler/scale G ==
  color : Inport
  scale : Inport  Port=2
  Product : Product  RndMeth=Zero
  Switch : Switch  Threshold=255
  scaled color : Outport
  wires:
  color/1 -> Product/2, Switch/2, Switch/3
  Switch/1 -> scaled color/1
  Product/1 -> Switch/1
  scale/1 -> Product/1

== system TSR_Scenario_005_cs/VISULightPostLight_Lightpost_5/scaler/scale R ==
  color : Inport
  scale : Inport  Port=2
  Product : Product  RndMeth=Zero
  Switch : Switch  Threshold=255
  scaled color : Outport
  wires:
  scale/1 -> Product/1
  Product/1 -> Switch/1
  Switch/1 -> scaled color/1
  color/1 -> Switch/3, Switch/2, Product/2

== system TSR_Scenario_005_cs/VISULightPostLight_Lightpost_54/scaler/scale B ==
  color : Inport
  scale : Inport  Port=2
  Product : Product  RndMeth=Zero
  Switch : Switch  Threshold=255
  scaled color : Outport
  wires:
  color/1 -> Product/2, Switch/2, Switch/3
  Switch/1 -> scaled color/1
  Product/1 -> Switch/1
  scale/1 -> Product/1

== system TSR_Scenario_005_cs/VISULightPostLight_Lightpost_54/scaler/scale G ==
  color : Inport
  scale : Inport  Port=2
  Product : Product  RndMeth=Zero
  Switch : Switch  Threshold=255
  scaled color : Outport
  wires:
  color/1 -> Product/2, Switch/2, Switch/3
  Switch/1 -> scaled color/1
  Product/1 -> Switch/1
  scale/1 -> Product/1

== system TSR_Scenario_005_cs/VISULightPostLight_Lightpost_54/scaler/scale R ==
  color : Inport
  scale : Inport  Port=2
  Product : Product  RndMeth=Zero
  Switch : Switch  Threshold=255
  scaled color : Outport
  wires:
  scale/1 -> Product/1
  Product/1 -> Switch/1
  Switch/1 -> scaled color/1
  color/1 -> Switch/3, Switch/2, Product/2

== system TSR_Scenario_005_cs/VISUWheels_Audi_A8_1/conv_RotWheelFL/RotMat2AxisAngle ==
  RotMat_Visu : Inport
  Data Type Conversion : DataTypeConversion
  Divide : Product  Inputs=*/
  Gain : Gain  Gain=2
  Mux : Mux  Inputs=3
  Mux1 : Mux  Inputs=2
  Saturation1 : Saturate  UpperLimit=3-0.001  LowerLimit=-1+0.001
  Trigonometric Function : Trigonometry
  U1 : Fcn  Expr=u[6]-u[8]
  U2 : Fcn  Expr=u[7]-u[3]
  U3 : Fcn  Expr=u[2]-u[4]
  U_Phi : Fcn  Expr=u[1]+u[5]+u[9]
  U_Phi1 : Fcn  Expr=acos((u(1)-1)/2)
  U_Phi2 : Fcn  Expr=acos((u(1)-1)/2)
  Angles_Visu : Outport
  wires:
  U_Phi2/1 -> Trigonometric Function/1
  Saturation1/1 -> U_Phi2/1
  U_Phi1/1 -> Data Type Conversion/1
  U_Phi/1 -> Saturation1/1, U_Phi1/1
  Divide/1 -> Mux1/1
  Mux1/1 -> Angles_Visu/1
  Gain/1 -> Divide/2
  Mux/1 -> Divide/1
  U3/1 -> Mux/3
  U2/1 -> Mux/2
  U1/1 -> Mux/1
  Trigonometric Function/1 -> Gain/1
  RotMat_Visu/1 -> U_Phi/1, U3/1, U2/1, U1/1
  Data Type Conversion/1 -> Mux1/2

== system TSR_Scenario_005_cs/VISUWheels_Audi_A8_1/conv_RotWheelFR/RotMat2AxisAngle ==
  RotMat_Visu : Inport
  Data Type Conversion : DataTypeConversion
  Divide : Product  Inputs=*/
  Gain : Gain  Gain=2
  Mux : Mux  Inputs=3
  Mux1 : Mux  Inputs=2
  Saturation1 : Saturate  UpperLimit=3-0.001  LowerLimit=-1+0.001
  Trigonometric Function : Trigonometry
  U1 : Fcn  Expr=u[6]-u[8]
  U2 : Fcn  Expr=u[7]-u[3]
  U3 : Fcn  Expr=u[2]-u[4]
  U_Phi : Fcn  Expr=u[1]+u[5]+u[9]
  U_Phi1 : Fcn  Expr=acos((u(1)-1)/2)
  U_Phi2 : Fcn  Expr=acos((u(1)-1)/2)
  Angles_Visu : Outport
  wires:
  Data Type Conversion/1 -> Mux1/2
  RotMat_Visu/1 -> U1/1, U2/1, U3/1, U_Phi/1
  Trigonometric Function/1 -> Gain/1
  U1/1 -> Mux/1
  U2/1 -> Mux/2
  U3/1 -> Mux/3
  Mux/1 -> Divide/1
  Gain/1 -> Divide/2
  Mux1/1 -> Angles_Visu/1
  Divide/1 -> Mux1/1
  U_Phi/1 -> U_Phi1/1, Saturation1/1
  U_Phi1/1 -> Data Type Conversion/1
  Saturation1/1 -> U_Phi2/1
  U_Phi2/1 -> Trigonometric Function/1

== system TSR_Scenario_005_cs/VISUWheels_Audi_A8_1/conv_RotWheelRL/RotMat2AxisAngle ==
  RotMat_Visu : Inport
  Data Type Conversion : DataTypeConversion
  Divide : Product  Inputs=*/
  Gain : Gain  Gain=2
  Mux : Mux  Inputs=3
  Mux1 : Mux  Inputs=2
  Saturation1 : Saturate  UpperLimit=3-0.001  LowerLimit=-1+0.001
  Trigonometric Function : Trigonometry
  U1 : Fcn  Expr=u[6]-u[8]
  U2 : Fcn  Expr=u[7]-u[3]
  U3 : Fcn  Expr=u[2]-u[4]
  U_Phi : Fcn  Expr=u[1]+u[5]+u[9]
  U_Phi1 : Fcn  Expr=acos((u(1)-1)/2)
  U_Phi2 : Fcn  Expr=acos((u(1)-1)/2)
  Angles_Visu : Outport
  wires:
  Data Type Conversion/1 -> Mux1/2
  RotMat_Visu/1 -> U1/1, U2/1, U3/1, U_Phi/1
  Trigonometric Function/1 -> Gain/1
  U1/1 -> Mux/1
  U2/1 -> Mux/2
  U3/1 -> Mux/3
  Mux/1 -> Divide/1
  Gain/1 -> Divide/2
  Mux1/1 -> Angles_Visu/1
  Divide/1 -> Mux1/1
  U_Phi/1 -> U_Phi1/1, Saturation1/1
  U_Phi1/1 -> Data Type Conversion/1
  Saturation1/1 -> U_Phi2/1
  U_Phi2/1 -> Trigonometric Function/1

== system TSR_Scenario_005_cs/VISUWheels_Audi_A8_1/conv_RotWheelRR/RotMat2AxisAngle ==
  RotMat_Visu : Inport
  Data Type Conversion : DataTypeConversion
  Divide : Product  Inputs=*/
  Gain : Gain  Gain=2
  Mux : Mux  Inputs=3
  Mux1 : Mux  Inputs=2
  Saturation1 : Saturate  UpperLimit=3-0.001  LowerLimit=-1+0.001
  Trigonometric Function : Trigonometry
  U1 : Fcn  Expr=u[6]-u[8]
  U2 : Fcn  Expr=u[7]-u[3]
  U3 : Fcn  Expr=u[2]-u[4]
  U_Phi : Fcn  Expr=u[1]+u[5]+u[9]
  U_Phi1 : Fcn  Expr=acos((u(1)-1)/2)
  U_Phi2 : Fcn  Expr=acos((u(1)-1)/2)
  Angles_Visu : Outport
  wires:
  Data Type Conversion/1 -> Mux1/2
  RotMat_Visu/1 -> U1/1, U2/1, U3/1, U_Phi/1
  Trigonometric Function/1 -> Gain/1
  U1/1 -> Mux/1
  U2/1 -> Mux/2
  U3/1 -> Mux/3
  Mux/1 -> Divide/1
  Gain/1 -> Divide/2
  Mux1/1 -> Angles_Visu/1
  Divide/1 -> Mux1/1
  U_Phi/1 -> U_Phi1/1, Saturation1/1
  U_Phi1/1 -> Data Type Conversion/1
  Saturation1/1 -> U_Phi2/1
  U_Phi2/1 -> Trigonometric Function/1

== system TSR_Scenario_005_cs/VISUWheels_Audi_A8_1/conv_TransWheelFL/pos_ini_Visu ==
  Constant : Constant  Value=x_ini_VRML
  Constant1 : Constant  Value=y_ini_VRML
  Constant2 : Constant  Value=z_ini_VRML
  Mux1 : Mux  Inputs=3
  pos_ini_Visu : Outport
  wires:
  Constant2/1 -> Mux1/3
  Constant1/1 -> Mux1/2
  Mux1/1 -> pos_ini_Visu/1
  Constant/1 -> Mux1/1

== system TSR_Scenario_005_cs/VISUWheels_Audi_A8_1/conv_TransWheelFL/transISO2Visu ==
  transISO : Inport
  Demux : Demux  Outputs=3
  trans_x_Visu : Outport
  trans_y_Visu : Outport  Port=2
  trans_z_Visu : Outport  Port=3
  wires:
  Demux/3 -> trans_z_Visu/1
  transISO/1 -> Demux/1
  Demux/1 -> trans_x_Visu/1
  Demux/2 -> trans_y_Visu/1  "rot_y"

== system TSR_Scenario_005_cs/VISUWheels_Audi_A8_1/conv_TransWheelFR/pos_ini_Visu ==
  Constant : Constant  Value=x_ini_VRML
  Constant1 : Constant  Value=y_ini_VRML
  Constant2 : Constant  Value=z_ini_VRML
  Mux1 : Mux  Inputs=3
  pos_ini_Visu : Outport
  wires:
  Constant/1 -> Mux1/1
  Mux1/1 -> pos_ini_Visu/1
  Constant1/1 -> Mux1/2
  Constant2/1 -> Mux1/3

== system TSR_Scenario_005_cs/VISUWheels_Audi_A8_1/conv_TransWheelFR/transISO2Visu ==
  transISO : Inport
  Demux : Demux  Outputs=3
  trans_x_Visu : Outport
  trans_y_Visu : Outport  Port=2
  trans_z_Visu : Outport  Port=3
  wires:
  Demux/3 -> trans_z_Visu/1
  transISO/1 -> Demux/1
  Demux/1 -> trans_x_Visu/1
  Demux/2 -> trans_y_Visu/1  "rot_y"

== system TSR_Scenario_005_cs/VISUWheels_Audi_A8_1/conv_TransWheelRL/pos_ini_Visu ==
  Constant : Constant  Value=x_ini_VRML
  Constant1 : Constant  Value=y_ini_VRML
  Constant2 : Constant  Value=z_ini_VRML
  Mux1 : Mux  Inputs=3
  pos_ini_Visu : Outport
  wires:
  Constant/1 -> Mux1/1
  Mux1/1 -> pos_ini_Visu/1
  Constant1/1 -> Mux1/2
  Constant2/1 -> Mux1/3

== system TSR_Scenario_005_cs/VISUWheels_Audi_A8_1/conv_TransWheelRL/transISO2Visu ==
  transISO : Inport
  Demux : Demux  Outputs=3
  trans_x_Visu : Outport
  trans_y_Visu : Outport  Port=2
  trans_z_Visu : Outport  Port=3
  wires:
  Demux/3 -> trans_z_Visu/1
  transISO/1 -> Demux/1
  Demux/1 -> trans_x_Visu/1
  Demux/2 -> trans_y_Visu/1  "rot_y"

== system TSR_Scenario_005_cs/VISUWheels_Audi_A8_1/conv_TransWheelRR/pos_ini_Visu ==
  Constant : Constant  Value=x_ini_VRML
  Constant1 : Constant  Value=y_ini_VRML
  Constant2 : Constant  Value=z_ini_VRML
  Mux1 : Mux  Inputs=3
  pos_ini_Visu : Outport
  wires:
  Constant/1 -> Mux1/1
  Mux1/1 -> pos_ini_Visu/1
  Constant1/1 -> Mux1/2
  Constant2/1 -> Mux1/3

== system TSR_Scenario_005_cs/VISUWheels_Audi_A8_1/conv_TransWheelRR/transISO2Visu ==
  transISO : Inport
  Demux : Demux  Outputs=3
  trans_x_Visu : Outport
  trans_y_Visu : Outport  Port=2
  trans_z_Visu : Outport  Port=3
  wires:
  Demux/3 -> trans_z_Visu/1
  transISO/1 -> Demux/1
  Demux/1 -> trans_x_Visu/1
  Demux/2 -> trans_y_Visu/1  "rot_y"
